simulink model "ClimaMgrForAdjmtOfSp"  (R2022b)
settings: Solver=FixedStepDiscrete  StartTime=0.0  StopTime=10.0  FixedStep=1  RelTol=1e-3
settings Model: Creator=blue.ge  LastModifiedBy=blue.ge
settings DataIOCC: SaveFormat=StructureWithTime
settings OptimizationCC: DefaultParameterBehavior=Inlined  EnhancedBackFolding=on  PassReuseOutputArgsAs=Individual arguments  ZeroExternalMemoryAtStartup=off  ZeroInternalMemoryAtStartup=off  OptimizeBlockOrder=speed  DifferentSizesBufferReuse=on  OptimizationCustomize=off  EfficientTunableParamExpr=on
settings DebuggingCC: ParameterTunabilityLossMsg=error
settings ModelReferenceCC: ModelReferenceNumInstancesAllowed=Single
settings SFSimCC: MATLABDynamicMemAlloc=off
settings RTWCC: Description=AUTOSAR  SystemTargetFile=autosar.tlc  GenCodeOnly=on  TemplateMakefile=ert_default_tmf
settings CodeAppCC: ForceParamTrailComments=on  IgnoreCustomStorageClasses=off  OperatorAnnotations=on  SimulinkDataObjDesc=on  SFDataObjDesc=on  InsertBlockDesc=on
settings STFCustomTargetCC: TargetLangStandard=C99 (ISO)  MultiwordLength=256  ParMdlRefBuildCompliant=on  CompOptLevelCompliant=on  IncludeMdlTerminateFcn=off  CombineOutputUpdateFcns=on  SuppressErrorStatus=on  ERTCustomFileBanners=on  SupportNonFinite=off  SupportComplex=off  SupportContinuousTime=off  SupportNonInlinedSFcns=off  RemoveResetFunc=on  MATLABClassNameForMDSCustomization=Simulink.SoftwareTarget.ERTCustomization  AutosarCompliant=on  UseToolchainInfoCompliant=on  LookupTableObjectStructAxisOrder=2,1,3,4,...  LUTObjectStructOrderEvenSpacing=Size,Table,Breakpoints  SystemTargetFile=autosar.tlc
settings ERTTargetCC: TargetLangStandard=C99 (ISO)  ConcurrentExecutionCompliant=off  IncludeMdlTerminateFcn=off  SuppressErrorStatus=on  SupportNonFinite=off  SupportComplex=off  ModelStepFunctionPrototypeControlCompliant=off  CPPClassGenCompliant=off  AutosarCompliant=on  LookupTableObjectStructAxisOrder=2,1,3,4,...  LUTObjectStructOrderEvenSpacing=Size,Table,Breakpoints  InlinedParameterPlacement=NonHierarchical  ERTCustomFileTemplate=example_file_process.tlc

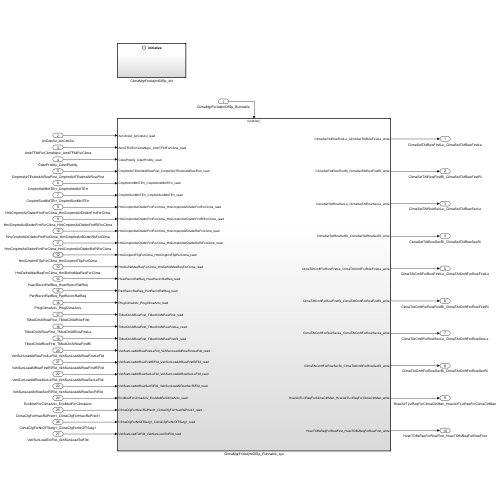
[thumbnail: Simulink's stored preview of the top-level diagram]
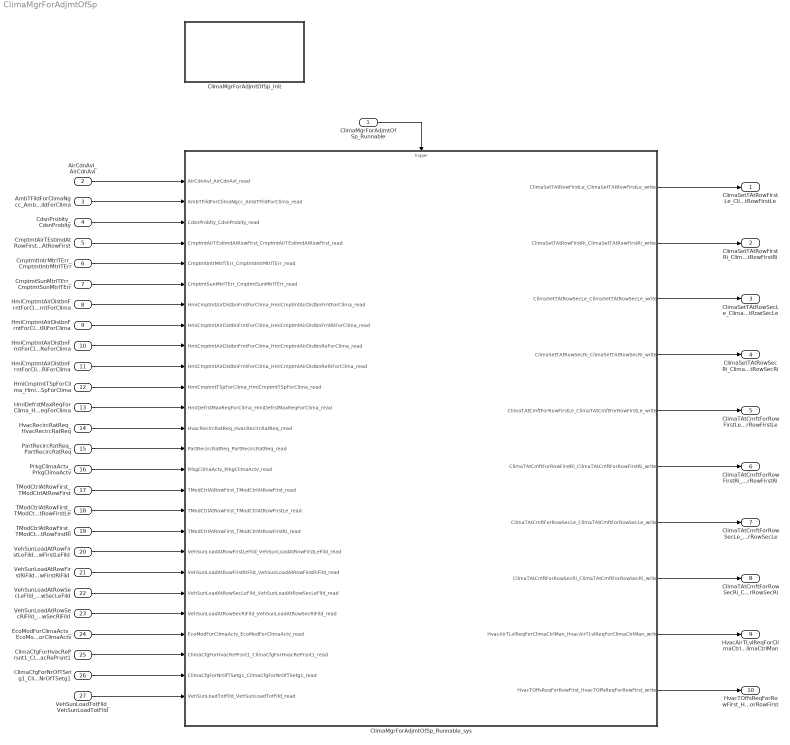
== system ClimaMgrForAdjmtOfSp ==
  ClimaMgrForAdjmtOfSp_Runnable : Inport  SampleTime=1  OutputFunctionCall=on
  AirCdnAvl_AirCdnAvl : Inport  Port=2  OutDataTypeStr=Boolean  PortDimensions=1
  AmbTFildForClimaNgcc_AmbTFildForClima : Inport  Port=3  OutDataTypeStr=T30  PortDimensions=1
  CdsnProblty_CdsnProblty : Inport  Port=4  OutDataTypeStr=Fac30  PortDimensions=1
  CmptmtAirTEstimdAtRowFirst_CmptmtAirTEstimdAtRowFirst : Inport  Port=5  OutDataTypeStr=T30  PortDimensions=1
  CmptmtIntrMtrlTErr_CmptmtIntrMtrlTErr : Inport  Port=6  OutDataTypeStr=T30  PortDimensions=1
  CmptmtSunMtrlTErr_CmptmtSunMtrlTErr : Inport  Port=7  OutDataTypeStr=T30  PortDimensions=1
  HmiCmptmtAirDistbnFrntForClima_HmiCmptmtAirDistbnFrntForClima : Inport  Port=8  OutDataTypeStr=Enum: HmiCmptmtAirDistbnFrnt  PortDimensions=1
  HmiCmptmtAirDistbnFrntForClima_HmiCmptmtAirDistbnFrntRiForClima : Inport  Port=9  OutDataTypeStr=Enum: HmiCmptmtAirDistbnFrnt  PortDimensions=1
  HmiCmptmtAirDistbnFrntForClima_HmiCmptmtAirDistbnReForClima : Inport  Port=10  OutDataTypeStr=Enum: HmiCmptmtAirDistbnFrnt  PortDimensions=1
  HmiCmptmtAirDistbnFrntForClima_HmiCmptmtAirDistbnReRiForClima : Inport  Port=11  OutDataTypeStr=Enum: HmiCmptmtAirDistbnFrnt  PortDimensions=1
  HmiCmptmtTSpForClima_HmiCmptmtTSpForClima : Inport  Port=12  OutDataTypeStr=Bus: HmiCmptmtTSp  PortDimensions=1  BusOutputAsStruct=on
  HmiDefrstMaxReqForClima_HmiDefrstMaxReqForClima : Inport  Port=13  OutDataTypeStr=Enum: ActrReq  PortDimensions=1
  HvacRecircRatReq_HvacRecircRatReq : Inport  Port=14  OutDataTypeStr=Perc30  PortDimensions=1
  PartRecircRatReq_PartRecircRatReq : Inport  Port=15  OutDataTypeStr=Perc30  PortDimensions=1
  PrkgClimaActv_PrkgClimaActv : Inport  Port=16  OutDataTypeStr=Enum: PrkgClimaActv  PortDimensions=1
  TModCtrlAtRowFirst_TModCtrlAtRowFirst : Inport  Port=17  OutDataTypeStr=T31  PortDimensions=1
  TModCtrlAtRowFirst_TModCtrlAtRowFirstLe : Inport  Port=18  OutDataTypeStr=T31  PortDimensions=1
  TModCtrlAtRowFirst_TModCtrlAtRowFirstRi : Inport  Port=19  OutDataTypeStr=T31  PortDimensions=1
  VehSunLoadAtRowFirstLeFild_VehSunLoadAtRowFirstLeFild : Inport  Port=20  OutDataTypeStr=SunLoad30  PortDimensions=1
  VehSunLoadAtRowFirstRiFild_VehSunLoadAtRowFirstRiFild : Inport  Port=21  OutDataTypeStr=SunLoad30  PortDimensions=1
  VehSunLoadAtRowSecLeFild_VehSunLoadAtRowSecLeFild : Inport  Port=22  OutDataTypeStr=SunLoad30  PortDimensions=1
  VehSunLoadAtRowSecRiFild_VehSunLoadAtRowSecRiFild : Inport  Port=23  OutDataTypeStr=SunLoad30  PortDimensions=1
  EcoModForClimaActv_EcoModForClimaActv : Inport  Port=24  OutDataTypeStr=Enum: OnOff1  PortDimensions=1
  ClimaCfgForHvacRePrsnt1_ClimaCfgForHvacRePrsnt1 : Inport  Port=25  OutDataTypeStr=Enum: CarCfgForHvacRe  PortDimensions=1
  ClimaCfgForNrOfTSetg1_ClimaCfgForNrOfTSetg1 : Inport  Port=26  OutDataTypeStr=Enum: ClimaCfgForNrOfTSetg1  PortDimensions=1
  VehSunLoadTotFild_VehSunLoadTotFild : Inport  Port=27  OutDataTypeStr=SunLoad30  PortDimensions=1
  ClimaMgrForAdjmtOfSp_Init : Subsystem
  ClimaMgrForAdjmtOfSp_Runnable_sys : Subsystem
  ClimaSetTAtRowFirstLe_ClimaSetTAtRowFirstLe : Outport  OutDataTypeStr=T30  PortDimensions=1
  ClimaSetTAtRowFirstRi_ClimaSetTAtRowFirstRi : Outport  Port=2  OutDataTypeStr=T30  PortDimensions=1
  ClimaSetTAtRowSecLe_ClimaSetTAtRowSecLe : Outport  Port=3  OutDataTypeStr=T30  PortDimensions=1
  ClimaSetTAtRowSecRi_ClimaSetTAtRowSecRi : Outport  Port=4  OutDataTypeStr=T30  PortDimensions=1
  ClimaTAtCmftForRowFirstLe_ClimaTAtCmftForRowFirstLe : Outport  Port=5  OutDataTypeStr=T30  PortDimensions=1
  ClimaTAtCmftForRowFirstRi_ClimaTAtCmftForRowFirstRi : Outport  Port=6  OutDataTypeStr=T30  PortDimensions=1
  ClimaTAtCmftForRowSecLe_ClimaTAtCmftForRowSecLe : Outport  Port=7  OutDataTypeStr=T30  PortDimensions=1
  ClimaTAtCmftForRowSecRi_ClimaTAtCmftForRowSecRi : Outport  Port=8  OutDataTypeStr=T30  PortDimensions=1
  HvacAirTLvlReqForClimaCtrlMan_HvacAirTLvlReqForClimaCtrlMan : Outport  Port=9  OutDataTypeStr=Perc30  PortDimensions=1
  HvacTOffsReqForRowFirst_HvacTOffsReqForRowFirst : Outport  Port=10  OutDataTypeStr=T30  PortDimensions=1
  wires:
  AirCdnAvl_AirCdnAvl/1 -> ClimaMgrForAdjmtOfSp_Runnable_sys/1(AirCdnAvl_AirCdnAvl_read)
  AmbTFildForClimaNgcc_AmbTFildForClima/1 -> ClimaMgrForAdjmtOfSp_Runnable_sys/2(AmbTFildForClimaNgcc_AmbTFildForClima_read)
  CdsnProblty_CdsnProblty/1 -> ClimaMgrForAdjmtOfSp_Runnable_sys/3(CdsnProblty_CdsnProblty_read)
  CmptmtAirTEstimdAtRowFirst_CmptmtAirTEstimdAtRowFirst/1 -> ClimaMgrForAdjmtOfSp_Runnable_sys/4(CmptmtAirTEstimdAtRowFirst_CmptmtAirTEstimdAtRowFirst_read)
  CmptmtIntrMtrlTErr_CmptmtIntrMtrlTErr/1 -> ClimaMgrForAdjmtOfSp_Runnable_sys/5(CmptmtIntrMtrlTErr_CmptmtIntrMtrlTErr_read)
  CmptmtSunMtrlTErr_CmptmtSunMtrlTErr/1 -> ClimaMgrForAdjmtOfSp_Runnable_sys/6(CmptmtSunMtrlTErr_CmptmtSunMtrlTErr_read)
  HmiCmptmtAirDistbnFrntForClima_HmiCmptmtAirDistbnFrntForClima/1 -> ClimaMgrForAdjmtOfSp_Runnable_sys/7(HmiCmptmtAirDistbnFrntForClima_HmiCmptmtAirDistbnFrntForClima_read)
  HmiCmptmtAirDistbnFrntForClima_HmiCmptmtAirDistbnFrntRiForClima/1 -> ClimaMgrForAdjmtOfSp_Runnable_sys/8(HmiCmptmtAirDistbnFrntForClima_HmiCmptmtAirDistbnFrntRiForClima_read)
  HmiCmptmtAirDistbnFrntForClima_HmiCmptmtAirDistbnReForClima/1 -> ClimaMgrForAdjmtOfSp_Runnable_sys/9(HmiCmptmtAirDistbnFrntForClima_HmiCmptmtAirDistbnReForClima_read)
  HmiCmptmtAirDistbnFrntForClima_HmiCmptmtAirDistbnReRiForClima/1 -> ClimaMgrForAdjmtOfSp_Runnable_sys/10(HmiCmptmtAirDistbnFrntForClima_HmiCmptmtAirDistbnReRiForClima_read)
  HmiCmptmtTSpForClima_HmiCmptmtTSpForClima/1 -> ClimaMgrForAdjmtOfSp_Runnable_sys/11(HmiCmptmtTSpForClima_HmiCmptmtTSpForClima_read)
  HmiDefrstMaxReqForClima_HmiDefrstMaxReqForClima/1 -> ClimaMgrForAdjmtOfSp_Runnable_sys/12(HmiDefrstMaxReqForClima_HmiDefrstMaxReqForClima_read)
  HvacRecircRatReq_HvacRecircRatReq/1 -> ClimaMgrForAdjmtOfSp_Runnable_sys/13(HvacRecircRatReq_HvacRecircRatReq_read)
  PartRecircRatReq_PartRecircRatReq/1 -> ClimaMgrForAdjmtOfSp_Runnable_sys/14(PartRecircRatReq_PartRecircRatReq_read)
  PrkgClimaActv_PrkgClimaActv/1 -> ClimaMgrForAdjmtOfSp_Runnable_sys/15(PrkgClimaActv_PrkgClimaActv_read)
  TModCtrlAtRowFirst_TModCtrlAtRowFirst/1 -> ClimaMgrForAdjmtOfSp_Runnable_sys/16(TModCtrlAtRowFirst_TModCtrlAtRowFirst_read)
  TModCtrlAtRowFirst_TModCtrlAtRowFirstLe/1 -> ClimaMgrForAdjmtOfSp_Runnable_sys/17(TModCtrlAtRowFirst_TModCtrlAtRowFirstLe_read)
  TModCtrlAtRowFirst_TModCtrlAtRowFirstRi/1 -> ClimaMgrForAdjmtOfSp_Runnable_sys/18(TModCtrlAtRowFirst_TModCtrlAtRowFirstRi_read)
  VehSunLoadAtRowFirstLeFild_VehSunLoadAtRowFirstLeFild/1 -> ClimaMgrForAdjmtOfSp_Runnable_sys/19(VehSunLoadAtRowFirstLeFild_VehSunLoadAtRowFirstLeFild_read)
  VehSunLoadAtRowFirstRiFild_VehSunLoadAtRowFirstRiFild/1 -> ClimaMgrForAdjmtOfSp_Runnable_sys/20(VehSunLoadAtRowFirstRiFild_VehSunLoadAtRowFirstRiFild_read)
  VehSunLoadAtRowSecLeFild_VehSunLoadAtRowSecLeFild/1 -> ClimaMgrForAdjmtOfSp_Runnable_sys/21(VehSunLoadAtRowSecLeFild_VehSunLoadAtRowSecLeFild_read)
  VehSunLoadAtRowSecRiFild_VehSunLoadAtRowSecRiFild/1 -> ClimaMgrForAdjmtOfSp_Runnable_sys/22(VehSunLoadAtRowSecRiFild_VehSunLoadAtRowSecRiFild_read)
  EcoModForClimaActv_EcoModForClimaActv/1 -> ClimaMgrForAdjmtOfSp_Runnable_sys/23(EcoModForClimaActv_EcoModForClimaActv_read)
  ClimaCfgForHvacRePrsnt1_ClimaCfgForHvacRePrsnt1/1 -> ClimaMgrForAdjmtOfSp_Runnable_sys/24(ClimaCfgForHvacRePrsnt1_ClimaCfgForHvacRePrsnt1_read)
  ClimaCfgForNrOfTSetg1_ClimaCfgForNrOfTSetg1/1 -> ClimaMgrForAdjmtOfSp_Runnable_sys/25(ClimaCfgForNrOfTSetg1_ClimaCfgForNrOfTSetg1_read)
  VehSunLoadTotFild_VehSunLoadTotFild/1 -> ClimaMgrForAdjmtOfSp_Runnable_sys/26(VehSunLoadTotFild_VehSunLoadTotFild_read)
  ClimaMgrForAdjmtOfSp_Runnable_sys/1(ClimaSetTAtRowFirstLe_ClimaSetTAtRowFirstLe_write) -> ClimaSetTAtRowFirstLe_ClimaSetTAtRowFirstLe/1
  ClimaMgrForAdjmtOfSp_Runnable_sys/2(ClimaSetTAtRowFirstRi_ClimaSetTAtRowFirstRi_write) -> ClimaSetTAtRowFirstRi_ClimaSetTAtRowFirstRi/1
  ClimaMgrForAdjmtOfSp_Runnable_sys/3(ClimaSetTAtRowSecLe_ClimaSetTAtRowSecLe_write) -> ClimaSetTAtRowSecLe_ClimaSetTAtRowSecLe/1
  ClimaMgrForAdjmtOfSp_Runnable_sys/4(ClimaSetTAtRowSecRi_ClimaSetTAtRowSecRi_write) -> ClimaSetTAtRowSecRi_ClimaSetTAtRowSecRi/1
  ClimaMgrForAdjmtOfSp_Runnable_sys/5(ClimaTAtCmftForRowFirstLe_ClimaTAtCmftForRowFirstLe_write) -> ClimaTAtCmftForRowFirstLe_ClimaTAtCmftForRowFirstLe/1
  ClimaMgrForAdjmtOfSp_Runnable_sys/6(ClimaTAtCmftForRowFirstRi_ClimaTAtCmftForRowFirstRi_write) -> ClimaTAtCmftForRowFirstRi_ClimaTAtCmftForRowFirstRi/1
  ClimaMgrForAdjmtOfSp_Runnable_sys/7(ClimaTAtCmftForRowSecLe_ClimaTAtCmftForRowSecLe_write) -> ClimaTAtCmftForRowSecLe_ClimaTAtCmftForRowSecLe/1
  ClimaMgrForAdjmtOfSp_Runnable_sys/8(ClimaTAtCmftForRowSecRi_ClimaTAtCmftForRowSecRi_write) -> ClimaTAtCmftForRowSecRi_ClimaTAtCmftForRowSecRi/1
  ClimaMgrForAdjmtOfSp_Runnable_sys/9(HvacAirTLvlReqForClimaCtrlMan_HvacAirTLvlReqForClimaCtrlMan_write) -> HvacAirTLvlReqForClimaCtrlMan_HvacAirTLvlReqForClimaCtrlMan/1
  ClimaMgrForAdjmtOfSp_Runnable_sys/10(HvacTOffsReqForRowFirst_HvacTOffsReqForRowFirst_write) -> HvacTOffsReqForRowFirst_HvacTOffsReqForRowFirst/1
  ClimaMgrForAdjmtOfSp_Runnable/1 -> ClimaMgrForAdjmtOfSp_Runnable_sys/Trigger

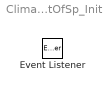
== system ClimaMgrForAdjmtOfSp/ClimaMgrForAdjmtOfSp_Init ==
  Event Listener : EventListener  EventName=reset

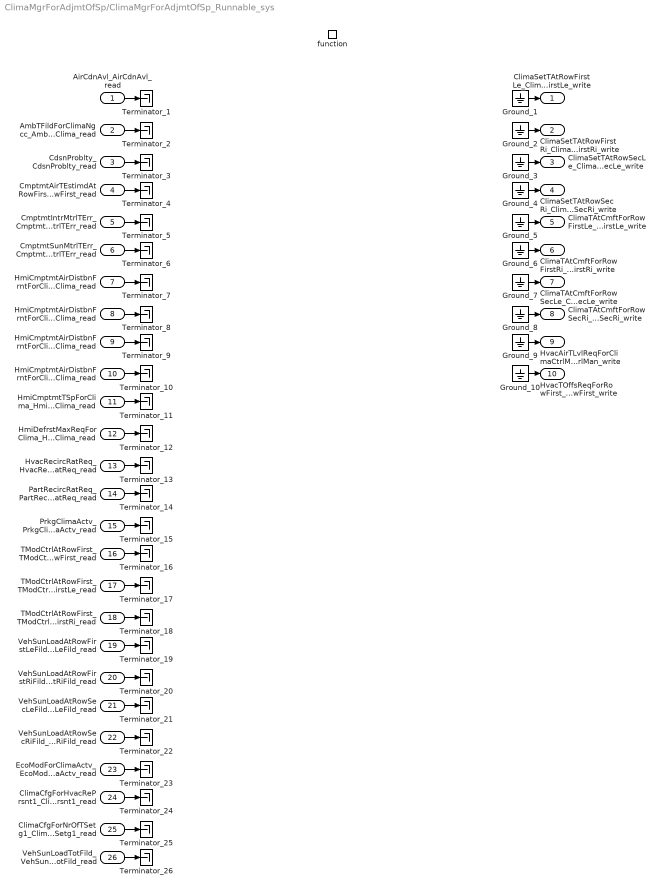
== system ClimaMgrForAdjmtOfSp/ClimaMgrForAdjmtOfSp_Runnable_sys ==
  AirCdnAvl_AirCdnAvl_read : Inport
  AmbTFildForClimaNgcc_AmbTFildForClima_read : Inport  Port=2
  CdsnProblty_CdsnProblty_read : Inport  Port=3
  CmptmtAirTEstimdAtRowFirst_CmptmtAirTEstimdAtRowFirst_read : Inport  Port=4
  CmptmtIntrMtrlTErr_CmptmtIntrMtrlTErr_read : Inport  Port=5
  CmptmtSunMtrlTErr_CmptmtSunMtrlTErr_read : Inport  Port=6
  HmiCmptmtAirDistbnFrntForClima_HmiCmptmtAirDistbnFrntForClima_read : Inport  Port=7
  HmiCmptmtAirDistbnFrntForClima_HmiCmptmtAirDistbnFrntRiForClima_read : Inport  Port=8
  HmiCmptmtAirDistbnFrntForClima_HmiCmptmtAirDistbnReForClima_read : Inport  Port=9
  HmiCmptmtAirDistbnFrntForClima_HmiCmptmtAirDistbnReRiForClima_read : Inport  Port=10
  HmiCmptmtTSpForClima_HmiCmptmtTSpForClima_read : Inport  Port=11
  HmiDefrstMaxReqForClima_HmiDefrstMaxReqForClima_read : Inport  Port=12
  HvacRecircRatReq_HvacRecircRatReq_read : Inport  Port=13
  PartRecircRatReq_PartRecircRatReq_read : Inport  Port=14
  PrkgClimaActv_PrkgClimaActv_read : Inport  Port=15
  TModCtrlAtRowFirst_TModCtrlAtRowFirst_read : Inport  Port=16
  TModCtrlAtRowFirst_TModCtrlAtRowFirstLe_read : Inport  Port=17
  TModCtrlAtRowFirst_TModCtrlAtRowFirstRi_read : Inport  Port=18
  VehSunLoadAtRowFirstLeFild_VehSunLoadAtRowFirstLeFild_read : Inport  Port=19
  VehSunLoadAtRowFirstRiFild_VehSunLoadAtRowFirstRiFild_read : Inport  Port=20
  VehSunLoadAtRowSecLeFild_VehSunLoadAtRowSecLeFild_read : Inport  Port=21
  VehSunLoadAtRowSecRiFild_VehSunLoadAtRowSecRiFild_read : Inport  Port=22
  EcoModForClimaActv_EcoModForClimaActv_read : Inport  Port=23
  ClimaCfgForHvacRePrsnt1_ClimaCfgForHvacRePrsnt1_read : Inport  Port=24
  ClimaCfgForNrOfTSetg1_ClimaCfgForNrOfTSetg1_read : Inport  Port=25
  VehSunLoadTotFild_VehSunLoadTotFild_read : Inport  Port=26
  function : TriggerPort  TriggerType=function-call  VariantControl=(inherit)  SampleTimeType=periodic  SampleTime=-1
  Ground_{1..10} (10 alike) : Ground
  Terminator_{1..26} (26 alike) : Terminator
  ClimaSetTAtRowFirstLe_ClimaSetTAtRowFirstLe_write : Outport
      settings: EnsureOutportIsVirtual=on
  ClimaSetTAtRowFirstRi_ClimaSetTAtRowFirstRi_write : Outport  Port=2
      settings: EnsureOutportIsVirtual=on
  ClimaSetTAtRowSecLe_ClimaSetTAtRowSecLe_write : Outport  Port=3
      settings: EnsureOutportIsVirtual=on
  ClimaSetTAtRowSecRi_ClimaSetTAtRowSecRi_write : Outport  Port=4
      settings: EnsureOutportIsVirtual=on
  ClimaTAtCmftForRowFirstLe_ClimaTAtCmftForRowFirstLe_write : Outport  Port=5
      settings: EnsureOutportIsVirtual=on
  ClimaTAtCmftForRowFirstRi_ClimaTAtCmftForRowFirstRi_write : Outport  Port=6
      settings: EnsureOutportIsVirtual=on
  ClimaTAtCmftForRowSecLe_ClimaTAtCmftForRowSecLe_write : Outport  Port=7
      settings: EnsureOutportIsVirtual=on
  ClimaTAtCmftForRowSecRi_ClimaTAtCmftForRowSecRi_write : Outport  Port=8
      settings: EnsureOutportIsVirtual=on
  HvacAirTLvlReqForClimaCtrlMan_HvacAirTLvlReqForClimaCtrlMan_write : Outport  Port=9
      settings: EnsureOutportIsVirtual=on
  HvacTOffsReqForRowFirst_HvacTOffsReqForRowFirst_write : Outport  Port=10
      settings: EnsureOutportIsVirtual=on
  wires:
  AirCdnAvl_AirCdnAvl_read/1 -> Terminator_1/1
  AmbTFildForClimaNgcc_AmbTFildForClima_read/1 -> Terminator_2/1
  CdsnProblty_CdsnProblty_read/1 -> Terminator_3/1
  CmptmtAirTEstimdAtRowFirst_CmptmtAirTEstimdAtRowFirst_read/1 -> Terminator_4/1
  CmptmtIntrMtrlTErr_CmptmtIntrMtrlTErr_read/1 -> Terminator_5/1
  CmptmtSunMtrlTErr_CmptmtSunMtrlTErr_read/1 -> Terminator_6/1
  HmiCmptmtAirDistbnFrntForClima_HmiCmptmtAirDistbnFrntForClima_read/1 -> Terminator_7/1
  HmiCmptmtAirDistbnFrntForClima_HmiCmptmtAirDistbnFrntRiForClima_read/1 -> Terminator_8/1
  HmiCmptmtAirDistbnFrntForClima_HmiCmptmtAirDistbnReForClima_read/1 -> Terminator_9/1
  HmiCmptmtAirDistbnFrntForClima_HmiCmptmtAirDistbnReRiForClima_read/1 -> Terminator_10/1
  HmiCmptmtTSpForClima_HmiCmptmtTSpForClima_read/1 -> Terminator_11/1
  HmiDefrstMaxReqForClima_HmiDefrstMaxReqForClima_read/1 -> Terminator_12/1
  HvacRecircRatReq_HvacRecircRatReq_read/1 -> Terminator_13/1
  PartRecircRatReq_PartRecircRatReq_read/1 -> Terminator_14/1
  PrkgClimaActv_PrkgClimaActv_read/1 -> Terminator_15/1
  TModCtrlAtRowFirst_TModCtrlAtRowFirst_read/1 -> Terminator_16/1
  TModCtrlAtRowFirst_TModCtrlAtRowFirstLe_read/1 -> Terminator_17/1
  TModCtrlAtRowFirst_TModCtrlAtRowFirstRi_read/1 -> Terminator_18/1
  VehSunLoadAtRowFirstLeFild_VehSunLoadAtRowFirstLeFild_read/1 -> Terminator_19/1
  VehSunLoadAtRowFirstRiFild_VehSunLoadAtRowFirstRiFild_read/1 -> Terminator_20/1
  VehSunLoadAtRowSecLeFild_VehSunLoadAtRowSecLeFild_read/1 -> Terminator_21/1
  VehSunLoadAtRowSecRiFild_VehSunLoadAtRowSecRiFild_read/1 -> Terminator_22/1
  EcoModForClimaActv_EcoModForClimaActv_read/1 -> Terminator_23/1
  ClimaCfgForHvacRePrsnt1_ClimaCfgForHvacRePrsnt1_read/1 -> Terminator_24/1
  ClimaCfgForNrOfTSetg1_ClimaCfgForNrOfTSetg1_read/1 -> Terminator_25/1
  VehSunLoadTotFild_VehSunLoadTotFild_read/1 -> Terminator_26/1
  Ground_1/1 -> ClimaSetTAtRowFirstLe_ClimaSetTAtRowFirstLe_write/1
  Ground_2/1 -> ClimaSetTAtRowFirstRi_ClimaSetTAtRowFirstRi_write/1
  Ground_3/1 -> ClimaSetTAtRowSecLe_ClimaSetTAtRowSecLe_write/1
  Ground_4/1 -> ClimaSetTAtRowSecRi_ClimaSetTAtRowSecRi_write/1
  Ground_5/1 -> ClimaTAtCmftForRowFirstLe_ClimaTAtCmftForRowFirstLe_write/1
  Ground_6/1 -> ClimaTAtCmftForRowFirstRi_ClimaTAtCmftForRowFirstRi_write/1
  Ground_7/1 -> ClimaTAtCmftForRowSecLe_ClimaTAtCmftForRowSecLe_write/1
  Ground_8/1 -> ClimaTAtCmftForRowSecRi_ClimaTAtCmftForRowSecRi_write/1
  Ground_9/1 -> HvacAirTLvlReqForClimaCtrlMan_HvacAirTLvlReqForClimaCtrlMan_write/1
  Ground_10/1 -> HvacTOffsReqForRowFirst_HvacTOffsReqForRowFirst_write/1
